simulink model "HvacMdlAirTDistbnDynFildFrnt"  (R2022b)
settings: Solver=FixedStepDiscrete  StartTime=0.0  StopTime=10.0  FixedStep=0.2  RelTol=1e-3
(part 2 of 2)

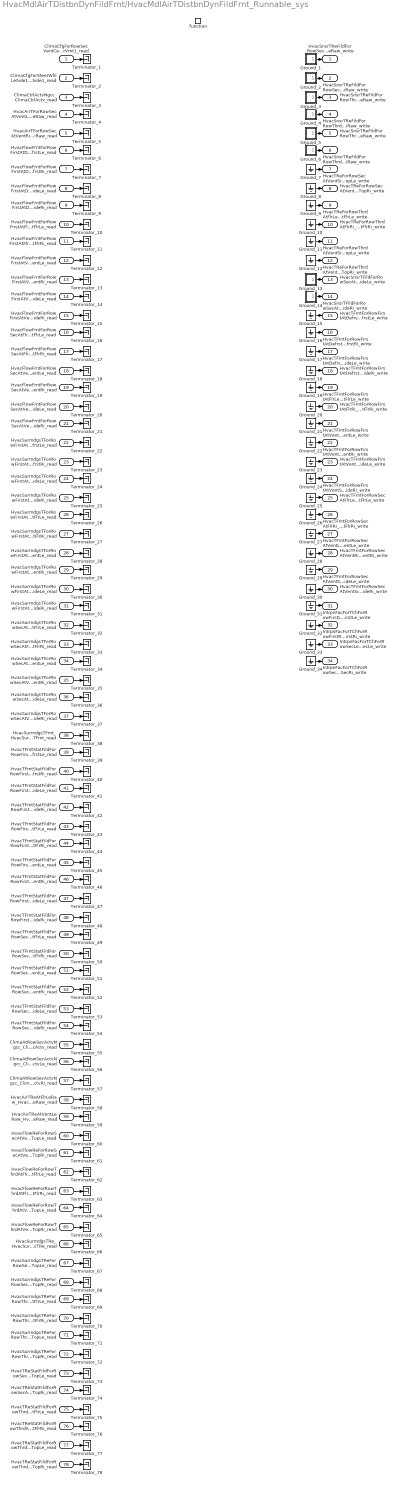
== system HvacMdlAirTDistbnDynFildFrnt/HvacMdlAirTDistbnDynFildFrnt_Runnable_sys ==
  ClimaCfgForRowSecVentCentrVrnt1_ClimaCfgForRowSecVentCentrVrnt1_read : Inport
  ClimaCfgForSteerWhlLeSide1_ClimaCfgForSteerWhlLeSide1_read : Inport  Port=2
  ClimaCtrlActvNgcc_ClimaCtrlActv_read : Inport  Port=3
  HvacAirTForRowSecAtVentLeRaw_HvacAirTForRowSecAtVentLeRaw_read : Inport  Port=4
  HvacAirTForRowSecAtVentRiRaw_HvacAirTForRowSecAtVentRiRaw_read : Inport  Port=5
  HvacFlowFrntForRowFirstAtDefrstLe_HvacFlowFrntForRowFirstAtDefrstLe_read : Inport  Port=6
  HvacFlowFrntForRowFirstAtDefrstRi_HvacFlowFrntForRowFirstAtDefrstRi_read : Inport  Port=7
  HvacFlowFrntForRowFirstAtDefrstSideLe_HvacFlowFrntForRowFirstAtDefrstSideLe_read : Inport  Port=8
  HvacFlowFrntForRowFirstAtDefrstSideRi_HvacFlowFrntForRowFirstAtDefrstSideRi_read : Inport  Port=9
  HvacFlowFrntForRowFirstAtFlrLe_HvacFlowFrntForRowFirstAtFlrLe_read : Inport  Port=10
  HvacFlowFrntForRowFirstAtFlrRi_HvacFlowFrntForRowFirstAtFlrRi_read : Inport  Port=11
  HvacFlowFrntForRowFirstAtVentLe_HvacFlowFrntForRowFirstAtVentLe_read : Inport  Port=12
  HvacFlowFrntForRowFirstAtVentRi_HvacFlowFrntForRowFirstAtVentRi_read : Inport  Port=13
  HvacFlowFrntForRowFirstAtVentSideLe_HvacFlowFrntForRowFirstAtVentSideLe_read : Inport  Port=14
  HvacFlowFrntForRowFirstAtVentSideRi_HvacFlowFrntForRowFirstAtVentSideRi_read : Inport  Port=15
  HvacFlowFrntForRowSecAtFlrLe_HvacFlowFrntForRowSecAtFlrLe_read : Inport  Port=16
  HvacFlowFrntForRowSecAtFlrRi_HvacFlowFrntForRowSecAtFlrRi_read : Inport  Port=17
  HvacFlowFrntForRowSecAtVentLe_HvacFlowFrntForRowSecAtVentLe_read : Inport  Port=18
  HvacFlowFrntForRowSecAtVentRi_HvacFlowFrntForRowSecAtVentRi_read : Inport  Port=19
  HvacFlowFrntForRowSecAtVentSideLe_HvacFlowFrntForRowSecAtVentSideLe_read : Inport  Port=20
  HvacFlowFrntForRowSecAtVentSideRi_HvacFlowFrntForRowSecAtVentSideRi_read : Inport  Port=21
  HvacSurrndgsTForRowFirstAtDefrstLe_HvacSurrndgsTForRowFirstAtDefrstLe_read : Inport  Port=22
  HvacSurrndgsTForRowFirstAtDefrstRi_HvacSurrndgsTForRowFirstAtDefrstRi_read : Inport  Port=23
  HvacSurrndgsTForRowFirstAtDefrstSideLe_HvacSurrndgsTForRowFirstAtDefrstSideLe_read : Inport  Port=24
  HvacSurrndgsTForRowFirstAtDefrstSideRi_HvacSurrndgsTForRowFirstAtDefrstSideRi_read : Inport  Port=25
  HvacSurrndgsTForRowFirstAtFlrLe_HvacSurrndgsTForRowFirstAtFlrLe_read : Inport  Port=26
  HvacSurrndgsTForRowFirstAtFlrRi_HvacSurrndgsTForRowFirstAtFlrRi_read : Inport  Port=27
  HvacSurrndgsTForRowFirstAtVentLe_HvacSurrndgsTForRowFirstAtVentLe_read : Inport  Port=28
  HvacSurrndgsTForRowFirstAtVentRi_HvacSurrndgsTForRowFirstAtVentRi_read : Inport  Port=29
  HvacSurrndgsTForRowFirstAtVentSideLe_HvacSurrndgsTForRowFirstAtVentSideLe_read : Inport  Port=30
  HvacSurrndgsTForRowFirstAtVentSideRi_HvacSurrndgsTForRowFirstAtVentSideRi_read : Inport  Port=31
  HvacSurrndgsTForRowSecAtFlrLe_HvacSurrndgsTForRowSecAtFlrLe_read : Inport  Port=32
  HvacSurrndgsTForRowSecAtFlrRi_HvacSurrndgsTForRowSecAtFlrRi_read : Inport  Port=33
  HvacSurrndgsTForRowSecAtVentLe_HvacSurrndgsTForRowSecAtVentLe_read : Inport  Port=34
  HvacSurrndgsTForRowSecAtVentRi_HvacSurrndgsTForRowSecAtVentRi_read : Inport  Port=35
  HvacSurrndgsTForRowSecAtVentSideLe_HvacSurrndgsTForRowSecAtVentSideLe_read : Inport  Port=36
  HvacSurrndgsTForRowSecAtVentSideRi_HvacSurrndgsTForRowSecAtVentSideRi_read : Inport  Port=37
  HvacSurrndgsTFrnt_HvacSurrndgsTFrnt_read : Inport  Port=38
  HvacTFrntStatFildForRowFirstAtDefrstLe_HvacTFrntStatFildForRowFirstAtDefrstLe_read : Inport  Port=39
  HvacTFrntStatFildForRowFirstAtDefrstRi_HvacTFrntStatFildForRowFirstAtDefrstRi_read : Inport  Port=40
  HvacTFrntStatFildForRowFirstAtDefrstSideLe_HvacTFrntStatFildForRowFirstAtDefrstSideLe_read : Inport  Port=41
  HvacTFrntStatFildForRowFirstAtDefrstSideRi_HvacTFrntStatFildForRowFirstAtDefrstSideRi_read : Inport  Port=42
  HvacTFrntStatFildForRowFirstAtFlrLe_HvacTFrntStatFildForRowFirstAtFlrLe_read : Inport  Port=43
  HvacTFrntStatFildForRowFirstAtFlrRi_HvacTFrntStatFildForRowFirstAtFlrRi_read : Inport  Port=44
  HvacTFrntStatFildForRowFirstAtVentLe_HvacTFrntStatFildForRowFirstAtVentLe_read : Inport  Port=45
  HvacTFrntStatFildForRowFirstAtVentRi_HvacTFrntStatFildForRowFirstAtVentRi_read : Inport  Port=46
  HvacTFrntStatFildForRowFirstAtVentSideLe_HvacTFrntStatFildForRowFirstAtVentSideLe_read : Inport  Port=47
  HvacTFrntStatFildForRowFirstAtVentSideRi_HvacTFrntStatFildForRowFirstAtVentSideRi_read : Inport  Port=48
  HvacTFrntStatFildForRowSecAtFlrLe_HvacTFrntStatFildForRowSecAtFlrLe_read : Inport  Port=49
  HvacTFrntStatFildForRowSecAtFlrRi_HvacTFrntStatFildForRowSecAtFlrRi_read : Inport  Port=50
  HvacTFrntStatFildForRowSecAtVentLe_HvacTFrntStatFildForRowSecAtVentLe_read : Inport  Port=51
  HvacTFrntStatFildForRowSecAtVentRi_HvacTFrntStatFildForRowSecAtVentRi_read : Inport  Port=52
  HvacTFrntStatFildForRowSecAtVentSideLe_HvacTFrntStatFildForRowSecAtVentSideLe_read : Inport  Port=53
  HvacTFrntStatFildForRowSecAtVentSideRi_HvacTFrntStatFildForRowSecAtVentSideRi_read : Inport  Port=54
  ClimaAtRowSecActvNgcc_ClimaAtRowSecActv_read : Inport  Port=55
  ClimaAtRowSecActvNgcc_ClimaAtRowSecActvLe_read : Inport  Port=56
  ClimaAtRowSecActvNgcc_ClimaAtRowSecActvRi_read : Inport  Port=57
  HvacAirTReAtFlrLeRaw_HvacAirTReAtFlrLeRaw_read : Inport  Port=58
  HvacAirTReAtVentLeRaw_HvacAirTReAtVentLeRaw_read : Inport  Port=59
  HvacFlowReForRowSecAtVentSideTopLe_HvacFlowReForRowSecAtVentSideTopLe_read : Inport  Port=60
  HvacFlowReForRowSecAtVentSideTopRi_HvacFlowReForRowSecAtVentSideTopRi_read : Inport  Port=61
  HvacFlowReForRowThrdAtFlrLe_HvacFlowReForRowThrdAtFlrLe_read : Inport  Port=62
  HvacFlowReForRowThrdAtFlrRi_HvacFlowReForRowThrdAtFlrRi_read : Inport  Port=63
  HvacFlowReForRowThrdAtVentSideTopLe_HvacFlowReForRowThrdAtVentSideTopLe_read : Inport  Port=64
  HvacFlowReForRowThrdAtVentSideTopRi_HvacFlowReForRowThrdAtVentSideTopRi_read : Inport  Port=65
  HvacSurrndgsTRe_HvacSurrndgsTRe_read : Inport  Port=66
  HvacSurrndgsTReForRowSecAtVentSideTopLe_HvacSurrndgsTReForRowSecAtVentSideTopLe_read : Inport  Port=67
  HvacSurrndgsTReForRowSecAtVentSideTopRi_HvacSurrndgsTReForRowSecAtVentSideTopRi_read : Inport  Port=68
  HvacSurrndgsTReForRowThrdAtFlrLe_HvacSurrndgsTReForRowThrdAtFlrLe_read : Inport  Port=69
  HvacSurrndgsTReForRowThrdAtFlrRi_HvacSurrndgsTReForRowThrdAtFlrRi_read : Inport  Port=70
  HvacSurrndgsTReForRowThrdAtVentSideTopLe_HvacSurrndgsTReForRowThrdAtVentSideTopLe_read : Inport  Port=71
  HvacSurrndgsTReForRowThrdAtVentSideTopRi_HvacSurrndgsTReForRowThrdAtVentSideTopRi_read : Inport  Port=72
  HvacTReStatFildForRowSecAtVentSideTopLe_HvacTReStatFildForRowSecAtVentSideTopLe_read : Inport  Port=73
  HvacTReStatFildForRowSecAtVentSideTopRi_HvacTReStatFildForRowSecAtVentSideTopRi_read : Inport  Port=74
  HvacTReStatFildForRowThrdAtFlrLe_HvacTReStatFildForRowThrdAtFlrLe_read : Inport  Port=75
  HvacTReStatFildForRowThrdAtFlrRi_HvacTReStatFildForRowThrdAtFlrRi_read : Inport  Port=76
  HvacTReStatFildForRowThrdAtVentSideTopLe_HvacTReStatFildForRowThrdAtVentSideTopLe_read : Inport  Port=77
  HvacTReStatFildForRowThrdAtVentSideTopRi_HvacTReStatFildForRowThrdAtVentSideTopRi_read : Inport  Port=78
  function : TriggerPort  TriggerType=function-call  VariantControl=(inherit)  SampleTimeType=periodic  SampleTime=-1
  Ground_1 : Subsystem
  Ground_{7..12,15..34} (26 alike) : Ground
  Ground_13 : Subsystem
  Ground_14 : Subsystem
  Ground_2 : Subsystem
  Ground_3 : Subsystem
  Ground_4 : Subsystem
  Ground_5 : Subsystem
  Ground_6 : Subsystem
  Terminator_{1..78} (78 alike) : Terminator
  HvacSnsrTReFildForRowSecAtVentSideTopLeRaw_HvacSnsrTReFildForRowSecAtVentSideTopLeRaw_write : Outport
      settings: EnsureOutportIsVirtual=on
  HvacSnsrTReFildForRowSecAtVentSideTopRiRaw_HvacSnsrTReFildForRowSecAtVentSideTopRiRaw_write : Outport  Port=2
      settings: EnsureOutportIsVirtual=on
  HvacSnsrTReFildForRowThrdAtFlrLeRaw_HvacSnsrTReFildForRowThrdAtFlrLeRaw_write : Outport  Port=3
      settings: EnsureOutportIsVirtual=on
  HvacSnsrTReFildForRowThrdAtFlrRiRaw_HvacSnsrTReFildForRowThrdAtFlrRiRaw_write : Outport  Port=4
      settings: EnsureOutportIsVirtual=on
  HvacSnsrTReFildForRowThrdAtVentSideTopLeRaw_HvacSnsrTReFildForRowThrdAtVentSideTopLeRaw_write : Outport  Port=5
      settings: EnsureOutportIsVirtual=on
  HvacSnsrTReFildForRowThrdAtVentSideTopRiRaw_HvacSnsrTReFildForRowThrdAtVentSideTopRiRaw_write : Outport  Port=6
      settings: EnsureOutportIsVirtual=on
  HvacTReForRowSecAtVentSideTopLe_HvacTReForRowSecAtVentSideTopLe_write : Outport  Port=7
      settings: EnsureOutportIsVirtual=on
  HvacTReForRowSecAtVentSideTopRi_HvacTReForRowSecAtVentSideTopRi_write : Outport  Port=8
      settings: EnsureOutportIsVirtual=on
  HvacTReForRowThrdAtFlrLe_HvacTReForRowThrdAtFlrLe_write : Outport  Port=9
      settings: EnsureOutportIsVirtual=on
  HvacTReForRowThrdAtFlrRi_HvacTReForRowThrdAtFlrRi_write : Outport  Port=10
      settings: EnsureOutportIsVirtual=on
  HvacTReForRowThrdAtVentSideTopLe_HvacTReForRowThrdAtVentSideTopLe_write : Outport  Port=11
      settings: EnsureOutportIsVirtual=on
  HvacTReForRowThrdAtVentSideTopRi_HvacTReForRowThrdAtVentSideTopRi_write : Outport  Port=12
      settings: EnsureOutportIsVirtual=on
  HvacSnsrTFildForRowSecAtVentSideLe_HvacSnsrTFildForRowSecAtVentSideLe_write : Outport  Port=13
      settings: EnsureOutportIsVirtual=on
  HvacSnsrTFildForRowSecAtVentSideRi_HvacSnsrTFildForRowSecAtVentSideRi_write : Outport  Port=14
      settings: EnsureOutportIsVirtual=on
  HvacTFrntForRowFirstAtDefrstLe_HvacTFrntForRowFirstAtDefrstLe_write : Outport  Port=15
      settings: EnsureOutportIsVirtual=on
  HvacTFrntForRowFirstAtDefrstRi_HvacTFrntForRowFirstAtDefrstRi_write : Outport  Port=16
      settings: EnsureOutportIsVirtual=on
  HvacTFrntForRowFirstAtDefrstSideLe_HvacTFrntForRowFirstAtDefrstSideLe_write : Outport  Port=17
      settings: EnsureOutportIsVirtual=on
  HvacTFrntForRowFirstAtDefrstSideRi_HvacTFrntForRowFirstAtDefrstSideRi_write : Outport  Port=18
      settings: EnsureOutportIsVirtual=on
  HvacTFrntForRowFirstAtFlrLe_HvacTFrntForRowFirstAtFlrLe_write : Outport  Port=19
      settings: EnsureOutportIsVirtual=on
  HvacTFrntForRowFirstAtFlrRi_HvacTFrntForRowFirstAtFlrRi_write : Outport  Port=20
      settings: EnsureOutportIsVirtual=on
  HvacTFrntForRowFirstAtVentLe_HvacTFrntForRowFirstAtVentLe_write : Outport  Port=21
      settings: EnsureOutportIsVirtual=on
  HvacTFrntForRowFirstAtVentRi_HvacTFrntForRowFirstAtVentRi_write : Outport  Port=22
      settings: EnsureOutportIsVirtual=on
  HvacTFrntForRowFirstAtVentSideLe_HvacTFrntForRowFirstAtVentSideLe_write : Outport  Port=23
      settings: EnsureOutportIsVirtual=on
  HvacTFrntForRowFirstAtVentSideRi_HvacTFrntForRowFirstAtVentSideRi_write : Outport  Port=24
      settings: EnsureOutportIsVirtual=on
  HvacTFrntForRowSecAtFlrLe_HvacTFrntForRowSecAtFlrLe_write : Outport  Port=25
      settings: EnsureOutportIsVirtual=on
  HvacTFrntForRowSecAtFlrRi_HvacTFrntForRowSecAtFlrRi_write : Outport  Port=26
      settings: EnsureOutportIsVirtual=on
  HvacTFrntForRowSecAtVentLe_HvacTFrntForRowSecAtVentLe_write : Outport  Port=27
      settings: EnsureOutportIsVirtual=on
  HvacTFrntForRowSecAtVentRi_HvacTFrntForRowSecAtVentRi_write : Outport  Port=28
      settings: EnsureOutportIsVirtual=on
  HvacTFrntForRowSecAtVentSideLe_HvacTFrntForRowSecAtVentSideLe_write : Outport  Port=29
      settings: EnsureOutportIsVirtual=on
  HvacTFrntForRowSecAtVentSideRi_HvacTFrntForRowSecAtVentSideRi_write : Outport  Port=30
      settings: EnsureOutportIsVirtual=on
  IntrpnFacForTChForRowFirstLe_IntrpnFacForTChForRowFirstLe_write : Outport  Port=31
      settings: EnsureOutportIsVirtual=on
  IntrpnFacForTChForRowFirstRi_IntrpnFacForTChForRowFirstRi_write : Outport  Port=32
      settings: EnsureOutportIsVirtual=on
  IntrpnFacForTChForRowSecLe_IntrpnFacForTChForRowSecLe_write : Outport  Port=33
      settings: EnsureOutportIsVirtual=on
  IntrpnFacForTChForRowSecRi_IntrpnFacForTChForRowSecRi_write : Outport  Port=34
      settings: EnsureOutportIsVirtual=on
  wires:
  ClimaCfgForRowSecVentCentrVrnt1_ClimaCfgForRowSecVentCentrVrnt1_read/1 -> Terminator_1/1
  ClimaCfgForSteerWhlLeSide1_ClimaCfgForSteerWhlLeSide1_read/1 -> Terminator_2/1
  ClimaCtrlActvNgcc_ClimaCtrlActv_read/1 -> Terminator_3/1
  HvacAirTForRowSecAtVentLeRaw_HvacAirTForRowSecAtVentLeRaw_read/1 -> Terminator_4/1
  HvacAirTForRowSecAtVentRiRaw_HvacAirTForRowSecAtVentRiRaw_read/1 -> Terminator_5/1
  HvacFlowFrntForRowFirstAtDefrstLe_HvacFlowFrntForRowFirstAtDefrstLe_read/1 -> Terminator_6/1
  HvacFlowFrntForRowFirstAtDefrstRi_HvacFlowFrntForRowFirstAtDefrstRi_read/1 -> Terminator_7/1
  HvacFlowFrntForRowFirstAtDefrstSideLe_HvacFlowFrntForRowFirstAtDefrstSideLe_read/1 -> Terminator_8/1
  HvacFlowFrntForRowFirstAtDefrstSideRi_HvacFlowFrntForRowFirstAtDefrstSideRi_read/1 -> Terminator_9/1
  HvacFlowFrntForRowFirstAtFlrLe_HvacFlowFrntForRowFirstAtFlrLe_read/1 -> Terminator_10/1
  HvacFlowFrntForRowFirstAtFlrRi_HvacFlowFrntForRowFirstAtFlrRi_read/1 -> Terminator_11/1
  HvacFlowFrntForRowFirstAtVentLe_HvacFlowFrntForRowFirstAtVentLe_read/1 -> Terminator_12/1
  HvacFlowFrntForRowFirstAtVentRi_HvacFlowFrntForRowFirstAtVentRi_read/1 -> Terminator_13/1
  HvacFlowFrntForRowFirstAtVentSideLe_HvacFlowFrntForRowFirstAtVentSideLe_read/1 -> Terminator_14/1
  HvacFlowFrntForRowFirstAtVentSideRi_HvacFlowFrntForRowFirstAtVentSideRi_read/1 -> Terminator_15/1
  HvacFlowFrntForRowSecAtFlrLe_HvacFlowFrntForRowSecAtFlrLe_read/1 -> Terminator_16/1
  HvacFlowFrntForRowSecAtFlrRi_HvacFlowFrntForRowSecAtFlrRi_read/1 -> Terminator_17/1
  HvacFlowFrntForRowSecAtVentLe_HvacFlowFrntForRowSecAtVentLe_read/1 -> Terminator_18/1
  HvacFlowFrntForRowSecAtVentRi_HvacFlowFrntForRowSecAtVentRi_read/1 -> Terminator_19/1
  HvacFlowFrntForRowSecAtVentSideLe_HvacFlowFrntForRowSecAtVentSideLe_read/1 -> Terminator_20/1
  HvacFlowFrntForRowSecAtVentSideRi_HvacFlowFrntForRowSecAtVentSideRi_read/1 -> Terminator_21/1
  HvacSurrndgsTForRowFirstAtDefrstLe_HvacSurrndgsTForRowFirstAtDefrstLe_read/1 -> Terminator_22/1
  HvacSurrndgsTForRowFirstAtDefrstRi_HvacSurrndgsTForRowFirstAtDefrstRi_read/1 -> Terminator_23/1
  HvacSurrndgsTForRowFirstAtDefrstSideLe_HvacSurrndgsTForRowFirstAtDefrstSideLe_read/1 -> Terminator_24/1
  HvacSurrndgsTForRowFirstAtDefrstSideRi_HvacSurrndgsTForRowFirstAtDefrstSideRi_read/1 -> Terminator_25/1
  HvacSurrndgsTForRowFirstAtFlrLe_HvacSurrndgsTForRowFirstAtFlrLe_read/1 -> Terminator_26/1
  HvacSurrndgsTForRowFirstAtFlrRi_HvacSurrndgsTForRowFirstAtFlrRi_read/1 -> Terminator_27/1
  HvacSurrndgsTForRowFirstAtVentLe_HvacSurrndgsTForRowFirstAtVentLe_read/1 -> Terminator_28/1
  HvacSurrndgsTForRowFirstAtVentRi_HvacSurrndgsTForRowFirstAtVentRi_read/1 -> Terminator_29/1
  HvacSurrndgsTForRowFirstAtVentSideLe_HvacSurrndgsTForRowFirstAtVentSideLe_read/1 -> Terminator_30/1
  HvacSurrndgsTForRowFirstAtVentSideRi_HvacSurrndgsTForRowFirstAtVentSideRi_read/1 -> Terminator_31/1
  HvacSurrndgsTForRowSecAtFlrLe_HvacSurrndgsTForRowSecAtFlrLe_read/1 -> Terminator_32/1
  HvacSurrndgsTForRowSecAtFlrRi_HvacSurrndgsTForRowSecAtFlrRi_read/1 -> Terminator_33/1
  HvacSurrndgsTForRowSecAtVentLe_HvacSurrndgsTForRowSecAtVentLe_read/1 -> Terminator_34/1
  HvacSurrndgsTForRowSecAtVentRi_HvacSurrndgsTForRowSecAtVentRi_read/1 -> Terminator_35/1
  HvacSurrndgsTForRowSecAtVentSideLe_HvacSurrndgsTForRowSecAtVentSideLe_read/1 -> Terminator_36/1
  HvacSurrndgsTForRowSecAtVentSideRi_HvacSurrndgsTForRowSecAtVentSideRi_read/1 -> Terminator_37/1
  HvacSurrndgsTFrnt_HvacSurrndgsTFrnt_read/1 -> Terminator_38/1
  HvacTFrntStatFildForRowFirstAtDefrstLe_HvacTFrntStatFildForRowFirstAtDefrstLe_read/1 -> Terminator_39/1
  HvacTFrntStatFildForRowFirstAtDefrstRi_HvacTFrntStatFildForRowFirstAtDefrstRi_read/1 -> Terminator_40/1
  HvacTFrntStatFildForRowFirstAtDefrstSideLe_HvacTFrntStatFildForRowFirstAtDefrstSideLe_read/1 -> Terminator_41/1
  HvacTFrntStatFildForRowFirstAtDefrstSideRi_HvacTFrntStatFildForRowFirstAtDefrstSideRi_read/1 -> Terminator_42/1
  HvacTFrntStatFildForRowFirstAtFlrLe_HvacTFrntStatFildForRowFirstAtFlrLe_read/1 -> Terminator_43/1
  HvacTFrntStatFildForRowFirstAtFlrRi_HvacTFrntStatFildForRowFirstAtFlrRi_read/1 -> Terminator_44/1
  HvacTFrntStatFildForRowFirstAtVentLe_HvacTFrntStatFildForRowFirstAtVentLe_read/1 -> Terminator_45/1
  HvacTFrntStatFildForRowFirstAtVentRi_HvacTFrntStatFildForRowFirstAtVentRi_read/1 -> Terminator_46/1
  HvacTFrntStatFildForRowFirstAtVentSideLe_HvacTFrntStatFildForRowFirstAtVentSideLe_read/1 -> Terminator_47/1
  HvacTFrntStatFildForRowFirstAtVentSideRi_HvacTFrntStatFildForRowFirstAtVentSideRi_read/1 -> Terminator_48/1
  HvacTFrntStatFildForRowSecAtFlrLe_HvacTFrntStatFildForRowSecAtFlrLe_read/1 -> Terminator_49/1
  HvacTFrntStatFildForRowSecAtFlrRi_HvacTFrntStatFildForRowSecAtFlrRi_read/1 -> Terminator_50/1
  HvacTFrntStatFildForRowSecAtVentLe_HvacTFrntStatFildForRowSecAtVentLe_read/1 -> Terminator_51/1
  HvacTFrntStatFildForRowSecAtVentRi_HvacTFrntStatFildForRowSecAtVentRi_read/1 -> Terminator_52/1
  HvacTFrntStatFildForRowSecAtVentSideLe_HvacTFrntStatFildForRowSecAtVentSideLe_read/1 -> Terminator_53/1
  HvacTFrntStatFildForRowSecAtVentSideRi_HvacTFrntStatFildForRowSecAtVentSideRi_read/1 -> Terminator_54/1
  ClimaAtRowSecActvNgcc_ClimaAtRowSecActv_read/1 -> Terminator_55/1
  ClimaAtRowSecActvNgcc_ClimaAtRowSecActvLe_read/1 -> Terminator_56/1
  ClimaAtRowSecActvNgcc_ClimaAtRowSecActvRi_read/1 -> Terminator_57/1
  HvacAirTReAtFlrLeRaw_HvacAirTReAtFlrLeRaw_read/1 -> Terminator_58/1
  HvacAirTReAtVentLeRaw_HvacAirTReAtVentLeRaw_read/1 -> Terminator_59/1
  HvacFlowReForRowSecAtVentSideTopLe_HvacFlowReForRowSecAtVentSideTopLe_read/1 -> Terminator_60/1
  HvacFlowReForRowSecAtVentSideTopRi_HvacFlowReForRowSecAtVentSideTopRi_read/1 -> Terminator_61/1
  HvacFlowReForRowThrdAtFlrLe_HvacFlowReForRowThrdAtFlrLe_read/1 -> Terminator_62/1
  HvacFlowReForRowThrdAtFlrRi_HvacFlowReForRowThrdAtFlrRi_read/1 -> Terminator_63/1
  HvacFlowReForRowThrdAtVentSideTopLe_HvacFlowReForRowThrdAtVentSideTopLe_read/1 -> Terminator_64/1
  HvacFlowReForRowThrdAtVentSideTopRi_HvacFlowReForRowThrdAtVentSideTopRi_read/1 -> Terminator_65/1
  HvacSurrndgsTRe_HvacSurrndgsTRe_read/1 -> Terminator_66/1
  HvacSurrndgsTReForRowSecAtVentSideTopLe_HvacSurrndgsTReForRowSecAtVentSideTopLe_read/1 -> Terminator_67/1
  HvacSurrndgsTReForRowSecAtVentSideTopRi_HvacSurrndgsTReForRowSecAtVentSideTopRi_read/1 -> Terminator_68/1
  HvacSurrndgsTReForRowThrdAtFlrLe_HvacSurrndgsTReForRowThrdAtFlrLe_read/1 -> Terminator_69/1
  HvacSurrndgsTReForRowThrdAtFlrRi_HvacSurrndgsTReForRowThrdAtFlrRi_read/1 -> Terminator_70/1
  HvacSurrndgsTReForRowThrdAtVentSideTopLe_HvacSurrndgsTReForRowThrdAtVentSideTopLe_read/1 -> Terminator_71/1
  HvacSurrndgsTReForRowThrdAtVentSideTopRi_HvacSurrndgsTReForRowThrdAtVentSideTopRi_read/1 -> Terminator_72/1
  HvacTReStatFildForRowSecAtVentSideTopLe_HvacTReStatFildForRowSecAtVentSideTopLe_read/1 -> Terminator_73/1
  HvacTReStatFildForRowSecAtVentSideTopRi_HvacTReStatFildForRowSecAtVentSideTopRi_read/1 -> Terminator_74/1
  HvacTReStatFildForRowThrdAtFlrLe_HvacTReStatFildForRowThrdAtFlrLe_read/1 -> Terminator_75/1
  HvacTReStatFildForRowThrdAtFlrRi_HvacTReStatFildForRowThrdAtFlrRi_read/1 -> Terminator_76/1
  HvacTReStatFildForRowThrdAtVentSideTopLe_HvacTReStatFildForRowThrdAtVentSideTopLe_read/1 -> Terminator_77/1
  HvacTReStatFildForRowThrdAtVentSideTopRi_HvacTReStatFildForRowThrdAtVentSideTopRi_read/1 -> Terminator_78/1
  Ground_1/1(Out1) -> HvacSnsrTReFildForRowSecAtVentSideTopLeRaw_HvacSnsrTReFildForRowSecAtVentSideTopLeRaw_write/1
  Ground_2/1(Out1) -> HvacSnsrTReFildForRowSecAtVentSideTopRiRaw_HvacSnsrTReFildForRowSecAtVentSideTopRiRaw_write/1
  Ground_3/1(Out1) -> HvacSnsrTReFildForRowThrdAtFlrLeRaw_HvacSnsrTReFildForRowThrdAtFlrLeRaw_write/1
  Ground_4/1(Out1) -> HvacSnsrTReFildForRowThrdAtFlrRiRaw_HvacSnsrTReFildForRowThrdAtFlrRiRaw_write/1
  Ground_5/1(Out1) -> HvacSnsrTReFildForRowThrdAtVentSideTopLeRaw_HvacSnsrTReFildForRowThrdAtVentSideTopLeRaw_write/1
  Ground_6/1(Out1) -> HvacSnsrTReFildForRowThrdAtVentSideTopRiRaw_HvacSnsrTReFildForRowThrdAtVentSideTopRiRaw_write/1
  Ground_7/1 -> HvacTReForRowSecAtVentSideTopLe_HvacTReForRowSecAtVentSideTopLe_write/1
  Ground_8/1 -> HvacTReForRowSecAtVentSideTopRi_HvacTReForRowSecAtVentSideTopRi_write/1
  Ground_9/1 -> HvacTReForRowThrdAtFlrLe_HvacTReForRowThrdAtFlrLe_write/1
  Ground_10/1 -> HvacTReForRowThrdAtFlrRi_HvacTReForRowThrdAtFlrRi_write/1
  Ground_11/1 -> HvacTReForRowThrdAtVentSideTopLe_HvacTReForRowThrdAtVentSideTopLe_write/1
  Ground_12/1 -> HvacTReForRowThrdAtVentSideTopRi_HvacTReForRowThrdAtVentSideTopRi_write/1
  Ground_13/1(Out1) -> HvacSnsrTFildForRowSecAtVentSideLe_HvacSnsrTFildForRowSecAtVentSideLe_write/1
  Ground_14/1(Out1) -> HvacSnsrTFildForRowSecAtVentSideRi_HvacSnsrTFildForRowSecAtVentSideRi_write/1
  Ground_15/1 -> HvacTFrntForRowFirstAtDefrstLe_HvacTFrntForRowFirstAtDefrstLe_write/1
  Ground_16/1 -> HvacTFrntForRowFirstAtDefrstRi_HvacTFrntForRowFirstAtDefrstRi_write/1
  Ground_17/1 -> HvacTFrntForRowFirstAtDefrstSideLe_HvacTFrntForRowFirstAtDefrstSideLe_write/1
  Ground_18/1 -> HvacTFrntForRowFirstAtDefrstSideRi_HvacTFrntForRowFirstAtDefrstSideRi_write/1
  Ground_19/1 -> HvacTFrntForRowFirstAtFlrLe_HvacTFrntForRowFirstAtFlrLe_write/1
  Ground_20/1 -> HvacTFrntForRowFirstAtFlrRi_HvacTFrntForRowFirstAtFlrRi_write/1
  Ground_21/1 -> HvacTFrntForRowFirstAtVentLe_HvacTFrntForRowFirstAtVentLe_write/1
  Ground_22/1 -> HvacTFrntForRowFirstAtVentRi_HvacTFrntForRowFirstAtVentRi_write/1
  Ground_23/1 -> HvacTFrntForRowFirstAtVentSideLe_HvacTFrntForRowFirstAtVentSideLe_write/1
  Ground_24/1 -> HvacTFrntForRowFirstAtVentSideRi_HvacTFrntForRowFirstAtVentSideRi_write/1
  Ground_25/1 -> HvacTFrntForRowSecAtFlrLe_HvacTFrntForRowSecAtFlrLe_write/1
  Ground_26/1 -> HvacTFrntForRowSecAtFlrRi_HvacTFrntForRowSecAtFlrRi_write/1
  Ground_27/1 -> HvacTFrntForRowSecAtVentLe_HvacTFrntForRowSecAtVentLe_write/1
  Ground_28/1 -> HvacTFrntForRowSecAtVentRi_HvacTFrntForRowSecAtVentRi_write/1
  Ground_29/1 -> HvacTFrntForRowSecAtVentSideLe_HvacTFrntForRowSecAtVentSideLe_write/1
  Ground_30/1 -> HvacTFrntForRowSecAtVentSideRi_HvacTFrntForRowSecAtVentSideRi_write/1
  Ground_31/1 -> IntrpnFacForTChForRowFirstLe_IntrpnFacForTChForRowFirstLe_write/1
  Ground_32/1 -> IntrpnFacForTChForRowFirstRi_IntrpnFacForTChForRowFirstRi_write/1
  Ground_33/1 -> IntrpnFacForTChForRowSecLe_IntrpnFacForTChForRowSecLe_write/1
  Ground_34/1 -> IntrpnFacForTChForRowSecRi_IntrpnFacForTChForRowSecRi_write/1

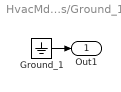
== system HvacMdlAirTDistbnDynFildFrnt/HvacMdlAirTDistbnDynFildFrnt_Runnable_sys/Ground_1 ==
  Ground_1 : Ground
  Out1 : Outport  OutDataTypeStr=Bus: HvacAirTReForRowSecAtVentSideTopLe  PortDimensions=1
  wires:
  Ground_1/1 -> Out1/1

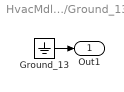
== system HvacMdlAirTDistbnDynFildFrnt/HvacMdlAirTDistbnDynFildFrnt_Runnable_sys/Ground_13 ==
  Ground_13 : Ground
  Out1 : Outport  OutDataTypeStr=Bus: HvacAirTForRowSecAtVentLe  PortDimensions=1
  wires:
  Ground_13/1 -> Out1/1

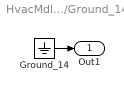
== system HvacMdlAirTDistbnDynFildFrnt/HvacMdlAirTDistbnDynFildFrnt_Runnable_sys/Ground_14 ==
  Ground_14 : Ground
  Out1 : Outport  OutDataTypeStr=Bus: HvacAirTForRowSecAtVentRi  PortDimensions=1
  wires:
  Ground_14/1 -> Out1/1

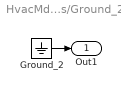
== system HvacMdlAirTDistbnDynFildFrnt/HvacMdlAirTDistbnDynFildFrnt_Runnable_sys/Ground_2 ==
  Ground_2 : Ground
  Out1 : Outport  OutDataTypeStr=Bus: HvacAirTReForRowSecAtVentSideTopRi  PortDimensions=1
  wires:
  Ground_2/1 -> Out1/1

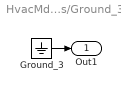
== system HvacMdlAirTDistbnDynFildFrnt/HvacMdlAirTDistbnDynFildFrnt_Runnable_sys/Ground_3 ==
  Ground_3 : Ground
  Out1 : Outport  OutDataTypeStr=Bus: HvacAirTReForRowThrdAtFlrLe  PortDimensions=1
  wires:
  Ground_3/1 -> Out1/1

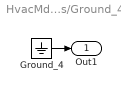
== system HvacMdlAirTDistbnDynFildFrnt/HvacMdlAirTDistbnDynFildFrnt_Runnable_sys/Ground_4 ==
  Ground_4 : Ground
  Out1 : Outport  OutDataTypeStr=Bus: HvacAirTReForRowThrdAtFlrRi  PortDimensions=1
  wires:
  Ground_4/1 -> Out1/1

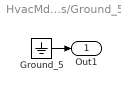
== system HvacMdlAirTDistbnDynFildFrnt/HvacMdlAirTDistbnDynFildFrnt_Runnable_sys/Ground_5 ==
  Ground_5 : Ground
  Out1 : Outport  OutDataTypeStr=Bus: HvacAirTReForRowThrdAtVentSideTopLe  PortDimensions=1
  wires:
  Ground_5/1 -> Out1/1

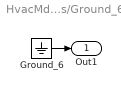
== system HvacMdlAirTDistbnDynFildFrnt/HvacMdlAirTDistbnDynFildFrnt_Runnable_sys/Ground_6 ==
  Ground_6 : Ground
  Out1 : Outport  OutDataTypeStr=Bus: HvacAirTReForRowThrdAtVentSideTopRi  PortDimensions=1
  wires:
  Ground_6/1 -> Out1/1
